simulink library "Microgrid_Library"  (R2020a)
settings: Solver=VariableStepAuto  StartTime=0.0  StopTime=10.0  RelTol=1e-3
libraries: simulink x6944, powerlib x1090, simulink_extras x40, powerlib_meascontrol x20
(part 1 of 77)
settings Model: Creator=burmesdani  LastModifiedBy=Daniel
settings DataIOCC: ReturnWorkspaceOutputs=off
settings DebuggingCC: ArithmeticOperatorsInVariantConditions=warning
settings SFSimCC: SimUseLocalCustomCode=off  CustomCodeUndefinedFunction=UseInterfaceOnly
settings RTWCC: RTWUseLocalCustomCode=off

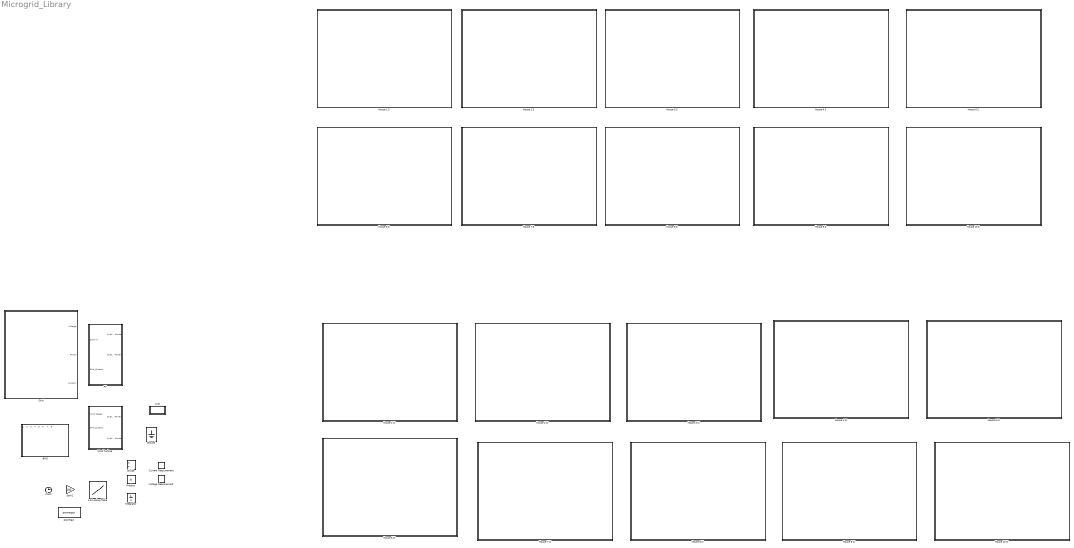
== system Microgrid_Library ==
  1-D Lookup Table : Lookup_n-D  NumberOfTableDimensions=1  BreakpointsForDimension1=[0 1 2 3 4 5 6 7 8 9 10 11 12 13 14 15 16 17 18 19 20 21 22 23]  Table=|  InternalRulePriority=Speed  RndMeth=Simplest  InputSameDT=on
      | [0
      | 0
      | 0
      | 0
      | 0
      | 0
      | 0
      | 0
      | 11
      | 36
      | 116
      | 198
      | 386
      | 372
      | 294
      | 178
      | 31
      | 0
      | 0
      | 0
      | 0
      | 0
      | 0
      | 0]
  BMS : Subsystem  mask{v=240, c=25}
      mask prompts: v "Voltage (V)"; c "Capacity (Ah)"
  Clock : Clock
  Current Measurement : powerlib/Measurements/Current Measurement  PhasorSimulation=off  OutputType=Complex  PSBequivalent=0
  Divide : Product  Inputs=*/
  Gain1 : Gain  Gain=100
  Grid : Subsystem  mask{v=240}
      mask prompts: v "Voltage"
      mask Display: image('power_pole_2.jpg');
  Ground : powerlib/Elements/Ground  LConnTagsString=a
  House 1 S : Subsystem  mask{V=240, t=60, K_L1_W=18, K_L1_On_1=0, K_L1_Off_1=27000, K_L1_On_2=73800, K_L1_Off_2=86400, K_L2_W=100, K_L2_On_1=61200, K_L2_Off_1=63000, Fridge_W=150, T_Initial=1, T_Low=1, T_High=2, Jug_W=2000, Jug_On_1=23400, Jug_On_2=61200, Jug_On_3=72000, Toaster_W=1100, Toaster_On_1=27000, Toaster_On_2=0, N_Elements=3, Oven_On_1=61200, Oven_Off_1=64800, Shower_On_a=69000, Shower_L_a=300, Shower_On_b=70200, Shower_L_b=300, Shower_On_c=76200, Shower_L_c=180, K_L3_W=40, K_L3_On_1=66600, K_L3_Off_1=69300, K_L3_On_2=79200, K_L3_Off_2=82800, HW_W=2000, M=135, D=0.488, L=1.335, Ti=7, Ta=20, T_Low_WH=57, T_High_WH=62.55, Clock_1_W=5, Lamp_1_W=25, L1_On_1=72600, L1_Off_1=82800, L1_On_2=0, L1_Off_2=0, K_L4_W=18, K_L4_On_1=72000, K_L4_Off_1=72600, Clock_2_W=3, Lamp_2_W=40, L2_On_1=25200, L2_Off_1=27000, L2_On_2=75600, L2_Off_2=82800, K_L5_W=40, K_L5_On_1=25200, K_L5_Off_1=27000, K_L5_On_2=75600, K_L5_Off_2=82800, Phone_C_1_W=5, Phone_C_1_On_a=0, Phone_C_1_Off_a=29700, Phone_C_1_On_b=79200, Phone_C_1_Off_b=86400, Phone_C_2_W=5, Phone_C_2_On_a=0, Phone_C_2_Off_a=29700, Phone_C_2_On_b=82800, Phone_C_2_Off_b=86400, Phone_C_3_W=5, Phone_C_3_On_a=0, Phone_C_3_Off_a=29700, Phone_C_3_On_b=82800, Phone_C_3_Off_b=86400, Laptop_C_1_W=65, Laptop_C_1_On_a=64800, Laptop_C_1_Off_a=72900, Laptop_C_2_W=60, Laptop_C_2_On_a=0, Laptop_C_2_Off_a=29700, Laptop_C_2_On_b=82800, Laptop_C_2_Off_b=86400, Lamp_3_W=40, L3_On_1=21600, L3_Off_1=27000, L3_On_2=72000, L3_Off_2=82800, K_L6_W=120, K_L6_On_1=68400, K_L6_Off_1=70200, Heater_W=2100, Heater_On_1=63660, Heater_Off_1=69240, Stereo_W=50, Stereo_On_1=57600, Stereo_Off_1=61500, TV_W=400, TV_On_1=21600, TV_Off_1=26400, TV_On_2=59400, TV_Off_2=79200}
      mask prompts: V "Voltage"; t "Time"; K_L1_W "Light_1_W"; K_L1_On_1 "Light_1_On_a"; K_L1_Off_1 "Light_1_Off_a"; K_L1_On_2 "Light_1_On_b"; K_L1_Off_2 "Light_1_Off_b"; K_L2_W "Light_2_W"; K_L2_On_1 "Light_2_On_a"; K_L2_Off_1 "Light_2_Off_a"; T_Initial "Fridge_Initial_Temp"; T_Low "Fridge_T_Low"; T_High "Fridge_T_High"; N_Elements "Oven_#_Elements"; Shower_L_a "Shower_Length_a"; Shower_L_b "Shower_Length_b"; Shower_L_c "Shower_Length_c"; K_L3_W "Light_3_W"; K_L3_On_1 "Light_3_On_a"; K_L3_Off_1 "Light_3_Off_a"; K_L3_On_2 "Light_3_On_b"; K_L3_Off_2 "Light_3_Off_b"; M "Mass of water"; D "HW_Diameter"; L "HW_Length"; Ti "Inlet Temp"; Ta "Ambient Temp"; L1_On_1 "Lamp_1_On_1"; L1_Off_1 "Lamp_1_Off_1"; L1_On_2 "Lamp_1_On_2"; L1_Off_2 "Lamp_1_Off_2"; K_L4_W "Light_4_W"; K_L4_On_1 "Light_4_On_a"; K_L4_Off_1 "Light_4_Off_a"; L2_On_1 "Lamp_2_On_1"; L2_Off_1 "Lamp_2_Off_1"; L2_On_2 "Lamp_2_On_2"; L2_Off_2 "Lamp_2_Off_2"; K_L5_W "Light_5_W"; K_L5_On_1 "Light_5_On_a"; K_L5_Off_1 "Light_5_Off_a"; K_L5_On_2 "Light_5_On_b"; K_L5_Off_2 "Light_5_Off_b"; L3_On_1 "Lamp_3_On_1"; L3_Off_1 "Lamp_3_Off_1"; L3_On_2 "Lamp_3_On_2"; L3_Off_2 "Lamp_3_Off_2"; K_L6_W "Light_6_W"; K_L6_On_1 "Light_6_On_a"; K_L6_Off_1 "Light_6_Off_a"
      mask DialogControl Container4 Prompt: House 1
      mask DialogControl Container10 Prompt: Kitchen
      mask DialogControl Container22 Prompt: Bathroom
      mask DialogControl Container20 Prompt: Bedroom 1
      mask DialogControl Container16 Prompt: Bedroom 2
      mask DialogControl Container11 Prompt: Lounge
      mask Display: image('house.jpg');
  House 1 W : Subsystem  mask{V=240, t=60, K_L1_W=18, K_L1_On_1=0, K_L1_Off_1=27000, K_L1_On_2=64800, K_L1_Off_2=86400, K_L2_W=100, K_L2_On_1=61200, K_L2_Off_1=63000, Fridge_W=150, T_Initial=1, T_Low=1, T_High=2, Jug_W=2000, Jug_On_1=23400, Jug_On_2=61200, Jug_On_3=72000, Toaster_W=1100, Toaster_On_1=27000, Toaster_On_2=0, N_Elements=3, Oven_On_1=61200, Oven_Off_1=64800, Shower_On_a=69000, Shower_L_a=300, Shower_On_b=70200, Shower_L_b=300, Shower_On_c=76200, Shower_L_c=180, K_L3_W=40, K_L3_On_1=66600, K_L3_Off_1=69300, K_L3_On_2=79200, K_L3_Off_2=82800, HW_W=2000, M=135, D=0.488, L=1.335, Ti=7, Ta=20, T_Low_WH=57, T_High_WH=62.55, Clock_1_W=5, Lamp_1_W=25, L1_On_1=70200, L1_Off_1=82800, L1_On_2=0, L1_Off_2=0, K_L4_W=18, K_L4_On_1=64800, K_L4_Off_1=70200, Clock_2_W=3, Lamp_2_W=40, L2_On_1=25200, L2_Off_1=27000, L2_On_2=75600, L2_Off_2=82800, K_L5_W=40, K_L5_On_1=25200, K_L5_Off_1=27000, K_L5_On_2=75600, K_L5_Off_2=82800, Phone_C_1_W=5, Phone_C_1_On_a=0, Phone_C_1_Off_a=29700, Phone_C_1_On_b=79200, Phone_C_1_Off_b=86400, Phone_C_2_W=5, Phone_C_2_On_a=0, Phone_C_2_Off_a=29700, Phone_C_2_On_b=82800, Phone_C_2_Off_b=86400, Phone_C_3_W=5, Phone_C_3_On_a=0, Phone_C_3_Off_a=29700, Phone_C_3_On_b=82800, Phone_C_3_Off_b=86400, Laptop_C_1_W=65, Laptop_C_1_On_a=64800, Laptop_C_1_Off_a=72900, Laptop_C_2_W=60, Laptop_C_2_On_a=0, Laptop_C_2_Off_a=29700, Laptop_C_2_On_b=82800, Laptop_C_2_Off_b=86400, Lamp_3_W=40, L3_On_1=21600, L3_Off_1=27000, L3_On_2=64800, L3_Off_2=68400, K_L6_W=120, K_L6_On_1=68400, K_L6_Off_1=70200, Heater_W=2100, Heater_On_1=63660, Heater_Off_1=69240, Stereo_W=50, Stereo_On_1=57600, Stereo_Off_1=61500, TV_W=400, TV_On_1=21600, TV_Off_1=26400, TV_On_2=59400, TV_Off_2=79200}
      mask prompts: V "Voltage"; t "Time"; K_L1_W "Light_1_W"; K_L1_On_1 "Light_1_On_a"; K_L1_Off_1 "Light_1_Off_a"; K_L1_On_2 "Light_1_On_b"; K_L1_Off_2 "Light_1_Off_b"; K_L2_W "Light_2_W"; K_L2_On_1 "Light_2_On_a"; K_L2_Off_1 "Light_2_Off_a"; T_Initial "Fridge_Initial_Temp"; T_Low "Fridge_T_Low"; T_High "Fridge_T_High"; N_Elements "Oven_#_Elements"; Shower_L_a "Shower_Length_a"; Shower_L_b "Shower_Length_b"; Shower_L_c "Shower_Length_c"; K_L3_W "Light_3_W"; K_L3_On_1 "Light_3_On_a"; K_L3_Off_1 "Light_3_Off_a"; K_L3_On_2 "Light_3_On_b"; K_L3_Off_2 "Light_3_Off_b"; M "Mass of water"; D "HW_Diameter"; L "HW_Length"; Ti "Inlet Temp"; Ta "Ambient Temp"; L1_On_1 "Lamp_1_On_1"; L1_Off_1 "Lamp_1_Off_1"; L1_On_2 "Lamp_1_On_2"; L1_Off_2 "Lamp_1_Off_2"; K_L4_W "Light_4_W"; K_L4_On_1 "Light_4_On_a"; K_L4_Off_1 "Light_4_Off_a"; L2_On_1 "Lamp_2_On_1"; L2_Off_1 "Lamp_2_Off_1"; L2_On_2 "Lamp_2_On_2"; L2_Off_2 "Lamp_2_Off_2"; K_L5_W "Light_5_W"; K_L5_On_1 "Light_5_On_a"; K_L5_Off_1 "Light_5_Off_a"; K_L5_On_2 "Light_5_On_b"; K_L5_Off_2 "Light_5_Off_b"; L3_On_1 "Lamp_3_On_1"; L3_Off_1 "Lamp_3_Off_1"; L3_On_2 "Lamp_3_On_2"; L3_Off_2 "Lamp_3_Off_2"; K_L6_W "Light_6_W"; K_L6_On_1 "Light_6_On_a"; K_L6_Off_1 "Light_6_Off_a"
      mask DialogControl Container4 Prompt: House 1
      mask DialogControl Container10 Prompt: Kitchen
      mask DialogControl Container22 Prompt: Bathroom
      mask DialogControl Container20 Prompt: Bedroom 1
      mask DialogControl Container16 Prompt: Bedroom 2
      mask DialogControl Container11 Prompt: Lounge
      mask Display: image('house.jpg');
  House 10 S : Subsystem  mask{V=240, t=60, K_L1_W=100, K_L1_On_1=25920, K_L1_Off_1=26940, K_L1_On_2=72120, K_L1_Off_2=75900, K_L2_W=40, K_L2_On_1=71820, K_L2_Off_1=73440, Fridge_W=150, T_Initial=1.2, T_Low=1, T_High=2.8, Jug_W=2000, Jug_On_1=26940, Jug_On_2=70980, Jug_On_3=0, Toaster_W=450, Toaster_On_1=25500, Toaster_On_2=0, N_Elements=2, Oven_On_1=66180, Oven_Off_1=67560, Shower_On_a=79680, Shower_L_a=420, Shower_On_b=80400, Shower_L_b=240, Shower_On_c=0, Shower_L_c=0, K_L3_W=24, K_L3_On_1=79200, K_L3_Off_1=80700, K_L3_On_2=0, K_L3_Off_2=0, HW_W=2000, M=135, D=0.488, L=1.335, Ti=7, Ta=20, T_Low_WH=58, T_High_WH=63.8, Clock_1_W=0, Lamp_1_W=1, L1_On_1=0, L1_Off_1=0, L1_On_2=0, L1_Off_2=0, K_L4_W=1, K_L4_On_1=0, K_L4_Off_1=0, Clock_2_W=5, Lamp_2_W=18, L2_On_1=25680, L2_Off_1=27600, L2_On_2=83940, L2_Off_2=85020, K_L5_W=40, K_L5_On_1=26400, K_L5_Off_1=27780, K_L5_On_2=81000, K_L5_Off_2=83940, Phone_C_1_W=7, Phone_C_1_On_a=0, Phone_C_1_Off_a=27300, Phone_C_1_On_b=79200, Phone_C_1_Off_b=86400, Phone_C_2_W=0, Phone_C_2_On_a=0, Phone_C_2_Off_a=0, Phone_C_2_On_b=0, Phone_C_2_Off_b=0, Phone_C_3_W=5, Phone_C_3_On_a=0, Phone_C_3_Off_a=27300, Phone_C_3_On_b=83940, Phone_C_3_Off_b=86400, Laptop_C_1_W=35, Laptop_C_1_On_a=63900, Laptop_C_1_Off_a=75600, Laptop_C_2_W=65, Laptop_C_2_On_a=0, Laptop_C_2_Off_a=21600, Laptop_C_2_On_b=66600, Laptop_C_2_Off_b=86400, Lamp_3_W=36, L3_On_1=27000, L3_Off_1=27600, L3_On_2=72600, L3_Off_2=81000, K_L6_W=24, K_L6_On_1=72000, K_L6_Off_1=75000, Heater_W=2000, Heater_On_1=65100, Heater_Off_1=69000, Stereo_W=1, Stereo_On_1=0, Stereo_Off_1=0, TV_W=200, TV_On_1=66300, TV_Off_1=83940, TV_On_2=0, TV_Off_2=0}
      mask prompts: V "Voltage"; t "Time"; K_L1_W "Light_1_W"; K_L1_On_1 "Light_1_On_a"; K_L1_Off_1 "Light_1_Off_a"; K_L1_On_2 "Light_1_On_b"; K_L1_Off_2 "Light_1_Off_b"; K_L2_W "Light_2_W"; K_L2_On_1 "Light_2_On_a"; K_L2_Off_1 "Light_2_Off_a"; T_Initial "Fridge_Initial_Temp"; T_Low "Fridge_T_Low"; T_High "Fridge_T_High"; N_Elements "Oven_#_Elements"; Shower_L_a "Shower_Length_a"; Shower_L_b "Shower_Length_b"; Shower_L_c "Shower_Length_c"; K_L3_W "Light_3_W"; K_L3_On_1 "Light_3_On_a"; K_L3_Off_1 "Light_3_Off_a"; K_L3_On_2 "Light_3_On_b"; K_L3_Off_2 "Light_3_Off_b"; M "Mass of water"; D "HW_Diameter"; L "HW_Length"; Ti "Inlet Temp"; Ta "Ambient Temp"; L1_On_1 "Lamp_1_On_1"; L1_Off_1 "Lamp_1_Off_1"; L1_On_2 "Lamp_1_On_2"; L1_Off_2 "Lamp_1_Off_2"; K_L4_W "Light_4_W"; K_L4_On_1 "Light_4_On_a"; K_L4_Off_1 "Light_4_Off_a"; L2_On_1 "Lamp_2_On_1"; L2_Off_1 "Lamp_2_Off_1"; L2_On_2 "Lamp_2_On_2"; L2_Off_2 "Lamp_2_Off_2"; K_L5_W "Light_5_W"; K_L5_On_1 "Light_5_On_a"; K_L5_Off_1 "Light_5_Off_a"; K_L5_On_2 "Light_5_On_b"; K_L5_Off_2 "Light_5_Off_b"; L3_On_1 "Lamp_3_On_1"; L3_Off_1 "Lamp_3_Off_1"; L3_On_2 "Lamp_3_On_2"; L3_Off_2 "Lamp_3_Off_2"; K_L6_W "Light_6_W"; K_L6_On_1 "Light_6_On_a"; K_L6_Off_1 "Light_6_Off_a"
      mask DialogControl Container4 Prompt: House 1
      mask DialogControl Container10 Prompt: Kitchen
      mask DialogControl Container22 Prompt: Bathroom
      mask DialogControl Container20 Prompt: Bedroom 1
      mask DialogControl Container16 Prompt: Bedroom 2
      mask DialogControl Container11 Prompt: Lounge
      mask Display: image('house.jpg');
  House 10 W : Subsystem  mask{V=240, t=60, K_L1_W=100, K_L1_On_1=25920, K_L1_Off_1=26940, K_L1_On_2=66120, K_L1_Off_2=69900, K_L2_W=40, K_L2_On_1=65820, K_L2_Off_1=67440, Fridge_W=150, T_Initial=1.2, T_Low=1, T_High=2.8, Jug_W=2000, Jug_On_1=26940, Jug_On_2=70980, Jug_On_3=0, Toaster_W=450, Toaster_On_1=25500, Toaster_On_2=0, N_Elements=2, Oven_On_1=66180, Oven_Off_1=67560, Shower_On_a=79680, Shower_L_a=420, Shower_On_b=80400, Shower_L_b=240, Shower_On_c=0, Shower_L_c=0, K_L3_W=24, K_L3_On_1=79200, K_L3_Off_1=80700, K_L3_On_2=0, K_L3_Off_2=0, HW_W=2000, M=135, D=0.488, L=1.335, Ti=7, Ta=20, T_Low_WH=58, T_High_WH=63.8, Clock_1_W=0, Lamp_1_W=1, L1_On_1=0, L1_Off_1=0, L1_On_2=0, L1_Off_2=0, K_L4_W=1, K_L4_On_1=0, K_L4_Off_1=0, Clock_2_W=5, Lamp_2_W=18, L2_On_1=25680, L2_Off_1=27600, L2_On_2=83940, L2_Off_2=85020, K_L5_W=40, K_L5_On_1=26400, K_L5_Off_1=27780, K_L5_On_2=81000, K_L5_Off_2=83940, Phone_C_1_W=7, Phone_C_1_On_a=0, Phone_C_1_Off_a=27300, Phone_C_1_On_b=79200, Phone_C_1_Off_b=86400, Phone_C_2_W=0, Phone_C_2_On_a=0, Phone_C_2_Off_a=0, Phone_C_2_On_b=0, Phone_C_2_Off_b=0, Phone_C_3_W=5, Phone_C_3_On_a=0, Phone_C_3_Off_a=27300, Phone_C_3_On_b=83940, Phone_C_3_Off_b=86400, Laptop_C_1_W=35, Laptop_C_1_On_a=63900, Laptop_C_1_Off_a=75600, Laptop_C_2_W=65, Laptop_C_2_On_a=0, Laptop_C_2_Off_a=21600, Laptop_C_2_On_b=66600, Laptop_C_2_Off_b=86400, Lamp_3_W=36, L3_On_1=27000, L3_Off_1=27600, L3_On_2=66600, L3_Off_2=81000, K_L6_W=24, K_L6_On_1=63000, K_L6_Off_1=66000, Heater_W=2000, Heater_On_1=65100, Heater_Off_1=69000, Stereo_W=1, Stereo_On_1=0, Stereo_Off_1=0, TV_W=200, TV_On_1=66300, TV_Off_1=83940, TV_On_2=0, TV_Off_2=0}
      mask prompts: V "Voltage"; t "Time"; K_L1_W "Light_1_W"; K_L1_On_1 "Light_1_On_a"; K_L1_Off_1 "Light_1_Off_a"; K_L1_On_2 "Light_1_On_b"; K_L1_Off_2 "Light_1_Off_b"; K_L2_W "Light_2_W"; K_L2_On_1 "Light_2_On_a"; K_L2_Off_1 "Light_2_Off_a"; T_Initial "Fridge_Initial_Temp"; T_Low "Fridge_T_Low"; T_High "Fridge_T_High"; N_Elements "Oven_#_Elements"; Shower_L_a "Shower_Length_a"; Shower_L_b "Shower_Length_b"; Shower_L_c "Shower_Length_c"; K_L3_W "Light_3_W"; K_L3_On_1 "Light_3_On_a"; K_L3_Off_1 "Light_3_Off_a"; K_L3_On_2 "Light_3_On_b"; K_L3_Off_2 "Light_3_Off_b"; M "Mass of water"; D "HW_Diameter"; L "HW_Length"; Ti "Inlet Temp"; Ta "Ambient Temp"; L1_On_1 "Lamp_1_On_1"; L1_Off_1 "Lamp_1_Off_1"; L1_On_2 "Lamp_1_On_2"; L1_Off_2 "Lamp_1_Off_2"; K_L4_W "Light_4_W"; K_L4_On_1 "Light_4_On_a"; K_L4_Off_1 "Light_4_Off_a"; L2_On_1 "Lamp_2_On_1"; L2_Off_1 "Lamp_2_Off_1"; L2_On_2 "Lamp_2_On_2"; L2_Off_2 "Lamp_2_Off_2"; K_L5_W "Light_5_W"; K_L5_On_1 "Light_5_On_a"; K_L5_Off_1 "Light_5_Off_a"; K_L5_On_2 "Light_5_On_b"; K_L5_Off_2 "Light_5_Off_b"; L3_On_1 "Lamp_3_On_1"; L3_Off_1 "Lamp_3_Off_1"; L3_On_2 "Lamp_3_On_2"; L3_Off_2 "Lamp_3_Off_2"; K_L6_W "Light_6_W"; K_L6_On_1 "Light_6_On_a"; K_L6_Off_1 "Light_6_Off_a"
      mask DialogControl Container4 Prompt: House 1
      mask DialogControl Container10 Prompt: Kitchen
      mask DialogControl Container22 Prompt: Bathroom
      mask DialogControl Container20 Prompt: Bedroom 1
      mask DialogControl Container16 Prompt: Bedroom 2
      mask DialogControl Container11 Prompt: Lounge
      mask Display: image('house.jpg');
  House 2 S : Subsystem  mask{V=240, t=60, K_L1_W=40, K_L1_On_1=75000, K_L1_Off_1=84600, K_L1_On_2=0, K_L1_Off_2=0, Dishwasher_W=1200, Dishwasher_On=72000, Fridge_W=150, T_Initial=1.9, T_Low=1, T_High=2.4, Jug_W=1500, Jug_On_1=25200, Jug_On_2=64200, Jug_On_3=0, Toaster_W=900, Toaster_On_1=23400, Toaster_On_2=27120, N_Elements=4, Oven_On_1=63600, Oven_Off_1=67200, Shower_On_a=21720, Shower_L_a=240, Shower_On_b=23640, Shower_L_b=300, Shower_On_c=0, Shower_L_c=0, K_L3_W=60, K_L3_On_1=21400, K_L3_Off_1=22140, K_L3_On_2=23580, K_L3_Off_2=24780, HW_W=2000, M=177, D=0.488, L=1.72, Ti=7, Ta=20, T_Low_WH=58, T_High_WH=61, Clock_1_W=5, Lamp_1_W=18, L1_On_1=79740, L1_Off_1=83040, L1_On_2=0, L1_Off_2=0, K_L4_W=24, K_L4_On_1=21000, K_L4_Off_1=27360, Clock_2_W=6, Lamp_2_W=24, L2_On_1=26820, L2_Off_1=29160, L2_On_2=73800, L2_Off_2=76800, K_L5_W=40, K_L5_On_1=28800, K_L5_Off_1=30240, K_L5_On_2=72300, K_L5_Off_2=83880, Phone_C_1_W=3, Phone_C_1_On_a=0, Phone_C_1_Off_a=35700, Phone_C_1_On_b=76800, Phone_C_1_Off_b=86400, Phone_C_2_W=7, Phone_C_2_On_a=0, Phone_C_2_Off_a=35760, Phone_C_2_On_b=76800, Phone_C_2_Off_b=86400, Phone_C_3_W=5, Phone_C_3_On_a=0, Phone_C_3_Off_a=35700, Phone_C_3_On_b=77040, Phone_C_3_Off_b=86400, Laptop_C_1_W=55, Laptop_C_1_On_a=76800, Laptop_C_1_Off_a=86400, Laptop_C_2_W=65, Laptop_C_2_On_a=0, Laptop_C_2_Off_a=28500, Laptop_C_2_On_b=77160, Laptop_C_2_Off_b=86400, Lamp_3_W=60, L3_On_1=21600, L3_Off_1=27000, L3_On_2=74760, L3_Off_2=83220, K_L6_W=60, K_L6_On_1=74400, K_L6_Off_1=76200, Heater_W=1800, Heater_On_1=62040, Heater_Off_1=68640, Stereo_W=100, Stereo_On_1=42480, Stereo_Off_1=58200, TV_W=500, TV_On_1=21900, TV_Off_1=26580, TV_On_2=63000, TV_Off_2=73200}
      mask prompts: V "Voltage"; t "Time"; K_L1_W "Light_1_W"; K_L1_On_1 "Light_1_On_a"; K_L1_Off_1 "Light_1_Off_a"; K_L1_On_2 "Light_1_On_b"; K_L1_Off_2 "Light_1_Off_b"; T_Initial "Fridge_Initial_Temp"; T_Low "Fridge_T_Low"; T_High "Fridge_T_High"; N_Elements "Oven_#_Elements"; Shower_L_a "Shower_Length_a"; Shower_L_b "Shower_Length_b"; Shower_L_c "Shower_Length_c"; K_L3_W "Light_3_W"; K_L3_On_1 "Light_3_On_a"; K_L3_Off_1 "Light_3_Off_a"; K_L3_On_2 "Light_3_On_b"; K_L3_Off_2 "Light_3_Off_b"; M "Mass of water"; D "HW_Diameter"; L "HW_Length"; Ti "Inlet Temp"; Ta "Ambient Temp"; L1_On_1 "Lamp_1_On_1"; L1_Off_1 "Lamp_1_Off_1"; L1_On_2 "Lamp_1_On_2"; L1_Off_2 "Lamp_1_Off_2"; K_L4_W "Light_4_W"; K_L4_On_1 "Light_4_On_a"; K_L4_Off_1 "Light_4_Off_a"; L2_On_1 "Lamp_2_On_1"; L2_Off_1 "Lamp_2_Off_1"; L2_On_2 "Lamp_2_On_2"; L2_Off_2 "Lamp_2_Off_2"; K_L5_W "Light_5_W"; K_L5_On_1 "Light_5_On_a"; K_L5_Off_1 "Light_5_Off_a"; K_L5_On_2 "Light_5_On_b"; K_L5_Off_2 "Light_5_Off_b"; L3_On_1 "Lamp_3_On_1"; L3_Off_1 "Lamp_3_Off_1"; L3_On_2 "Lamp_3_On_2"; L3_Off_2 "Lamp_3_Off_2"; K_L6_W "Light_6_W"; K_L6_On_1 "Light_6_On_a"; K_L6_Off_1 "Light_6_Off_a"
      mask DialogControl Container4 Prompt: House 1
      mask DialogControl Container10 Prompt: Kitchen
      mask DialogControl Container22 Prompt: Bathroom
      mask DialogControl Container20 Prompt: Bedroom 1
      mask DialogControl Container16 Prompt: Bedroom 2
      mask DialogControl Container11 Prompt: Lounge
      mask Display: image('house.jpg');
  House 2 W : Subsystem  mask{V=240, t=60, K_L1_W=40, K_L1_On_1=63000, K_L1_Off_1=72600, K_L1_On_2=0, K_L1_Off_2=0, Dishwasher_W=1200, Dishwasher_On=72000, Fridge_W=150, T_Initial=1.9, T_Low=1, T_High=2.4, Jug_W=1500, Jug_On_1=25200, Jug_On_2=64200, Jug_On_3=0, Toaster_W=900, Toaster_On_1=23400, Toaster_On_2=27120, N_Elements=4, Oven_On_1=63600, Oven_Off_1=67200, Shower_On_a=21720, Shower_L_a=240, Shower_On_b=23640, Shower_L_b=300, Shower_On_c=0, Shower_L_c=0, K_L3_W=60, K_L3_On_1=21480, K_L3_Off_1=22140, K_L3_On_2=23580, K_L3_Off_2=24780, HW_W=2000, M=177, D=0.488, L=1.72, Ti=7, Ta=20, T_Low_WH=58, T_High_WH=61, Clock_1_W=5, Lamp_1_W=18, L1_On_1=79740, L1_Off_1=83040, L1_On_2=0, L1_Off_2=0, K_L4_W=24, K_L4_On_1=21000, K_L4_Off_1=27360, Clock_2_W=6, Lamp_2_W=24, L2_On_1=26820, L2_Off_1=29160, L2_On_2=73800, L2_Off_2=76800, K_L5_W=40, K_L5_On_1=28800, K_L5_Off_1=30240, K_L5_On_2=72300, K_L5_Off_2=83880, Phone_C_1_W=3, Phone_C_1_On_a=0, Phone_C_1_Off_a=35700, Phone_C_1_On_b=76800, Phone_C_1_Off_b=86400, Phone_C_2_W=7, Phone_C_2_On_a=0, Phone_C_2_Off_a=35760, Phone_C_2_On_b=76800, Phone_C_2_Off_b=86400, Phone_C_3_W=5, Phone_C_3_On_a=0, Phone_C_3_Off_a=35700, Phone_C_3_On_b=77040, Phone_C_3_Off_b=86400, Laptop_C_1_W=55, Laptop_C_1_On_a=76800, Laptop_C_1_Off_a=86400, Laptop_C_2_W=65, Laptop_C_2_On_a=0, Laptop_C_2_Off_a=28500, Laptop_C_2_On_b=77160, Laptop_C_2_Off_b=86400, Lamp_3_W=60, L3_On_1=21600, L3_Off_1=27000, L3_On_2=62760, L3_Off_2=83220, K_L6_W=60, K_L6_On_1=68400, K_L6_Off_1=70200, Heater_W=1800, Heater_On_1=62040, Heater_Off_1=68640, Stereo_W=100, Stereo_On_1=42480, Stereo_Off_1=58200, TV_W=500, TV_On_1=21900, TV_Off_1=26580, TV_On_2=63000, TV_Off_2=73200}
      mask prompts: V "Voltage"; t "Time"; K_L1_W "Light_1_W"; K_L1_On_1 "Light_1_On_a"; K_L1_Off_1 "Light_1_Off_a"; K_L1_On_2 "Light_1_On_b"; K_L1_Off_2 "Light_1_Off_b"; T_Initial "Fridge_Initial_Temp"; T_Low "Fridge_T_Low"; T_High "Fridge_T_High"; N_Elements "Oven_#_Elements"; Shower_L_a "Shower_Length_a"; Shower_L_b "Shower_Length_b"; Shower_L_c "Shower_Length_c"; K_L3_W "Light_3_W"; K_L3_On_1 "Light_3_On_a"; K_L3_Off_1 "Light_3_Off_a"; K_L3_On_2 "Light_3_On_b"; K_L3_Off_2 "Light_3_Off_b"; M "Mass of water"; D "HW_Diameter"; L "HW_Length"; Ti "Inlet Temp"; Ta "Ambient Temp"; L1_On_1 "Lamp_1_On_1"; L1_Off_1 "Lamp_1_Off_1"; L1_On_2 "Lamp_1_On_2"; L1_Off_2 "Lamp_1_Off_2"; K_L4_W "Light_4_W"; K_L4_On_1 "Light_4_On_a"; K_L4_Off_1 "Light_4_Off_a"; L2_On_1 "Lamp_2_On_1"; L2_Off_1 "Lamp_2_Off_1"; L2_On_2 "Lamp_2_On_2"; L2_Off_2 "Lamp_2_Off_2"; K_L5_W "Light_5_W"; K_L5_On_1 "Light_5_On_a"; K_L5_Off_1 "Light_5_Off_a"; K_L5_On_2 "Light_5_On_b"; K_L5_Off_2 "Light_5_Off_b"; L3_On_1 "Lamp_3_On_1"; L3_Off_1 "Lamp_3_Off_1"; L3_On_2 "Lamp_3_On_2"; L3_Off_2 "Lamp_3_Off_2"; K_L6_W "Light_6_W"; K_L6_On_1 "Light_6_On_a"; K_L6_Off_1 "Light_6_Off_a"
      mask DialogControl Container4 Prompt: House 1
      mask DialogControl Container10 Prompt: Kitchen
      mask DialogControl Container22 Prompt: Bathroom
      mask DialogControl Container20 Prompt: Bedroom 1
      mask DialogControl Container16 Prompt: Bedroom 2
      mask DialogControl Container11 Prompt: Lounge
      mask Display: image('house.jpg');
  House 3 S : Subsystem  mask{V=240, t=60, K_L1_W=24, K_L1_On_1=21600, K_L1_Off_1=26580, K_L1_On_2=75000, K_L1_Off_2=79020, K_L2_W=60, K_L2_On_1=75000, K_L2_Off_1=80400, Fridge_W=200, T_Initial=1.5, T_Low=1.5, T_High=2.65, Jug_W=2000, Jug_On_1=21720, Jug_On_2=43560, Jug_On_3=73800, Toaster_W=1200, Toaster_On_1=25260, Toaster_On_2=28920, N_Elements=1, Oven_On_1=58680, Oven_Off_1=68760, Shower_On_a=70440, Shower_L_a=360, Shower_On_b=76740, Shower_L_b=180, Shower_On_c=81000, Shower_L_c=180, K_L3_W=80, K_L3_On_1=70200, K_L3_Off_1=70800, K_L3_On_2=76320, K_L3_Off_2=81300, HW_W=3000, M=250, D=0.58, L=1.56, Ti=7, Ta=20, T_Low_WH=59, T_High_WH=61, Clock_1_W=6, Lamp_1_W=18, L1_On_1=77400, L1_Off_1=81120, L1_On_2=0, L1_Off_2=0, K_L4_W=60, K_L4_On_1=75600, K_L4_Off_1=77400, Clock_2_W=4, Lamp_2_W=24, L2_On_1=84120, L2_Off_1=86880, L2_On_2=0, L2_Off_2=0, K_L5_W=40, K_L5_On_1=25320, K_L5_Off_1=28980, K_L5_On_2=81000, K_L5_Off_2=84180, Phone_C_1_W=7, Phone_C_1_On_a=0, Phone_C_1_Off_a=27300, Phone_C_1_On_b=79200, Phone_C_1_Off_b=86400, Phone_C_2_W=6, Phone_C_2_On_a=0, Phone_C_2_Off_a=27300, Phone_C_2_On_b=79200, Phone_C_2_Off_b=86400, Phone_C_3_W=5, Phone_C_3_On_a=0, Phone_C_3_Off_a=0, Phone_C_3_On_b=0, Phone_C_3_Off_b=0, Laptop_C_1_W=1, Laptop_C_1_On_a=0, Laptop_C_1_Off_a=0, Laptop_C_2_W=45, Laptop_C_2_On_a=0, Laptop_C_2_Off_a=6000, Laptop_C_2_On_b=66600, Laptop_C_2_Off_b=86400, Lamp_3_W=40, L3_On_1=24900, L3_Off_1=30600, L3_On_2=72000, L3_Off_2=81000, K_L6_W=60, K_L6_On_1=74760, K_L6_Off_1=77520, Heater_W=1500, Heater_On_1=64800, Heater_Off_1=78000, Stereo_W=1, Stereo_On_1=0, Stereo_Off_1=0, TV_W=150, TV_On_1=25200, TV_Off_1=28800, TV_On_2=72240, TV_Off_2=81000}
      mask prompts: V "Voltage"; t "Time"; K_L1_W "Light_1_W"; K_L1_On_1 "Light_1_On_a"; K_L1_Off_1 "Light_1_Off_a"; K_L1_On_2 "Light_1_On_b"; K_L1_Off_2 "Light_1_Off_b"; K_L2_W "Light_2_W"; K_L2_On_1 "Light_2_On_a"; K_L2_Off_1 "Light_2_Off_a"; T_Initial "Fridge_Initial_Temp"; T_Low "Fridge_T_Low"; T_High "Fridge_T_High"; N_Elements "Oven_#_Elements"; Shower_L_a "Shower_Length_a"; Shower_L_b "Shower_Length_b"; Shower_L_c "Shower_Length_c"; K_L3_W "Light_3_W"; K_L3_On_1 "Light_3_On_a"; K_L3_Off_1 "Light_3_Off_a"; K_L3_On_2 "Light_3_On_b"; K_L3_Off_2 "Light_3_Off_b"; M "Mass of water"; D "HW_Diameter"; L "HW_Length"; Ti "Inlet Temp"; Ta "Ambient Temp"; L1_On_1 "Lamp_1_On_1"; L1_Off_1 "Lamp_1_Off_1"; L1_On_2 "Lamp_1_On_2"; L1_Off_2 "Lamp_1_Off_2"; K_L4_W "Light_4_W"; K_L4_On_1 "Light_4_On_a"; K_L4_Off_1 "Light_4_Off_a"; L2_On_1 "Lamp_2_On_1"; L2_Off_1 "Lamp_2_Off_1"; L2_On_2 "Lamp_2_On_2"; L2_Off_2 "Lamp_2_Off_2"; K_L5_W "Light_5_W"; K_L5_On_1 "Light_5_On_a"; K_L5_Off_1 "Light_5_Off_a"; K_L5_On_2 "Light_5_On_b"; K_L5_Off_2 "Light_5_Off_b"; L3_On_1 "Lamp_3_On_1"; L3_Off_1 "Lamp_3_Off_1"; L3_On_2 "Lamp_3_On_2"; L3_Off_2 "Lamp_3_Off_2"; K_L6_W "Light_6_W"; K_L6_On_1 "Light_6_On_a"; K_L6_Off_1 "Light_6_Off_a"
      mask DialogControl Container4 Prompt: House 1
      mask DialogControl Container10 Prompt: Kitchen
      mask DialogControl Container22 Prompt: Bathroom
      mask DialogControl Container20 Prompt: Bedroom 1
      mask DialogControl Container16 Prompt: Bedroom 2
      mask DialogControl Container11 Prompt: Lounge
      mask Display: image('house.jpg');
  House 3 W : Subsystem  mask{V=240, t=60, K_L1_W=24, K_L1_On_1=21600, K_L1_Off_1=26580, K_L1_On_2=63000, K_L1_Off_2=68400, K_L2_W=60, K_L2_On_1=63000, K_L2_Off_1=68400, Fridge_W=200, T_Initial=1.5, T_Low=1.5, T_High=2.65, Jug_W=2000, Jug_On_1=21720, Jug_On_2=43560, Jug_On_3=73800, Toaster_W=1200, Toaster_On_1=25260, Toaster_On_2=28920, N_Elements=1, Oven_On_1=58680, Oven_Off_1=68760, Shower_On_a=70440, Shower_L_a=360, Shower_On_b=76740, Shower_L_b=180, Shower_On_c=81000, Shower_L_c=180, K_L3_W=80, K_L3_On_1=70200, K_L3_Off_1=70800, K_L3_On_2=76320, K_L3_Off_2=81300, HW_W=3000, M=250, D=0.58, L=1.56, Ti=7, Ta=20, T_Low_WH=59, T_High_WH=61, Clock_1_W=6, Lamp_1_W=18, L1_On_1=71400, L1_Off_1=81120, L1_On_2=0, L1_Off_2=0, K_L4_W=60, K_L4_On_1=69600, K_L4_Off_1=71400, Clock_2_W=4, Lamp_2_W=24, L2_On_1=84120, L2_Off_1=86880, L2_On_2=0, L2_Off_2=0, K_L5_W=40, K_L5_On_1=25320, K_L5_Off_1=28980, K_L5_On_2=81000, K_L5_Off_2=84180, Phone_C_1_W=7, Phone_C_1_On_a=0, Phone_C_1_Off_a=27300, Phone_C_1_On_b=79200, Phone_C_1_Off_b=86400, Phone_C_2_W=6, Phone_C_2_On_a=0, Phone_C_2_Off_a=27300, Phone_C_2_On_b=79200, Phone_C_2_Off_b=86400, Phone_C_3_W=5, Phone_C_3_On_a=0, Phone_C_3_Off_a=0, Phone_C_3_On_b=0, Phone_C_3_Off_b=0, Laptop_C_1_W=1, Laptop_C_1_On_a=0, Laptop_C_1_Off_a=0, Laptop_C_2_W=45, Laptop_C_2_On_a=0, Laptop_C_2_Off_a=6000, Laptop_C_2_On_b=66600, Laptop_C_2_Off_b=86400, Lamp_3_W=40, L3_On_1=24900, L3_Off_1=30600, L3_On_2=72000, L3_Off_2=81000, K_L6_W=60, K_L6_On_1=68760, K_L6_Off_1=71520, Heater_W=1500, Heater_On_1=64800, Heater_Off_1=78000, Stereo_W=1, Stereo_On_1=0, Stereo_Off_1=0, TV_W=150, TV_On_1=25200, TV_Off_1=28800, TV_On_2=72240, TV_Off_2=81000}
      mask prompts: V "Voltage"; t "Time"; K_L1_W "Light_1_W"; K_L1_On_1 "Light_1_On_a"; K_L1_Off_1 "Light_1_Off_a"; K_L1_On_2 "Light_1_On_b"; K_L1_Off_2 "Light_1_Off_b"; K_L2_W "Light_2_W"; K_L2_On_1 "Light_2_On_a"; K_L2_Off_1 "Light_2_Off_a"; T_Initial "Fridge_Initial_Temp"; T_Low "Fridge_T_Low"; T_High "Fridge_T_High"; N_Elements "Oven_#_Elements"; Shower_L_a "Shower_Length_a"; Shower_L_b "Shower_Length_b"; Shower_L_c "Shower_Length_c"; K_L3_W "Light_3_W"; K_L3_On_1 "Light_3_On_a"; K_L3_Off_1 "Light_3_Off_a"; K_L3_On_2 "Light_3_On_b"; K_L3_Off_2 "Light_3_Off_b"; M "Mass of water"; D "HW_Diameter"; L "HW_Length"; Ti "Inlet Temp"; Ta "Ambient Temp"; L1_On_1 "Lamp_1_On_1"; L1_Off_1 "Lamp_1_Off_1"; L1_On_2 "Lamp_1_On_2"; L1_Off_2 "Lamp_1_Off_2"; K_L4_W "Light_4_W"; K_L4_On_1 "Light_4_On_a"; K_L4_Off_1 "Light_4_Off_a"; L2_On_1 "Lamp_2_On_1"; L2_Off_1 "Lamp_2_Off_1"; L2_On_2 "Lamp_2_On_2"; L2_Off_2 "Lamp_2_Off_2"; K_L5_W "Light_5_W"; K_L5_On_1 "Light_5_On_a"; K_L5_Off_1 "Light_5_Off_a"; K_L5_On_2 "Light_5_On_b"; K_L5_Off_2 "Light_5_Off_b"; L3_On_1 "Lamp_3_On_1"; L3_Off_1 "Lamp_3_Off_1"; L3_On_2 "Lamp_3_On_2"; L3_Off_2 "Lamp_3_Off_2"; K_L6_W "Light_6_W"; K_L6_On_1 "Light_6_On_a"; K_L6_Off_1 "Light_6_Off_a"
      mask DialogControl Container4 Prompt: House 1
      mask DialogControl Container10 Prompt: Kitchen
      mask DialogControl Container22 Prompt: Bathroom
      mask DialogControl Container20 Prompt: Bedroom 1
      mask DialogControl Container16 Prompt: Bedroom 2
      mask DialogControl Container11 Prompt: Lounge
      mask Display: image('house.jpg');
  House 4 S : Subsystem  mask{V=240, t=60, K_L1_W=60, K_L1_On_1=75180, K_L1_Off_1=76200, K_L1_On_2=0, K_L1_Off_2=0, K_L2_W=1, K_L2_On_1=0, K_L2_Off_1=0, Fridge_W=150, T_Initial=1, T_Low=1, T_High=3.6, Jug_W=1800, Jug_On_1=23760, Jug_On_2=79800, Jug_On_3=0, Toaster_W=1000, Toaster_On_1=21600, Toaster_On_2=0, N_Elements=2, Oven_On_1=75240, Oven_Off_1=76140, Shower_On_a=20040, Shower_L_a=180, Shower_On_b=0, Shower_L_b=0, Shower_On_c=0, Shower_L_c=0, K_L3_W=40, K_L3_On_1=19800, K_L3_Off_1=20400, K_L3_On_2=0, K_L3_Off_2=0, HW_W=2000, M=88, D=0.488, L=0.955, Ti=7, Ta=20, T_Low_WH=55, T_High_WH=59.5, Clock_1_W=0, Lamp_1_W=1, L1_On_1=0, L1_Off_1=0, L1_On_2=0, L1_Off_2=0, K_L4_W=1, K_L4_On_1=0, K_L4_Off_1=0, Clock_2_W=4, Lamp_2_W=40, L2_On_1=19680, L2_Off_1=21600, L2_On_2=82800, L2_Off_2=84600, K_L5_W=1, K_L5_On_1=0, K_L5_Off_1=0, K_L5_On_2=0, K_L5_Off_2=0, Phone_C_1_W=5, Phone_C_1_On_a=0, Phone_C_1_Off_a=27300, Phone_C_1_On_b=79200, Phone_C_1_Off_b=86400, Phone_C_2_W=0, Phone_C_2_On_a=0, Phone_C_2_Off_a=0, Phone_C_2_On_b=0, Phone_C_2_Off_b=0, Phone_C_3_W=0, Phone_C_3_On_a=0, Phone_C_3_Off_a=0, Phone_C_3_On_b=0, Phone_C_3_Off_b=0, Laptop_C_1_W=35, Laptop_C_1_On_a=63900, Laptop_C_1_Off_a=75600, Laptop_C_2_W=65, Laptop_C_2_On_a=0, Laptop_C_2_Off_a=21600, Laptop_C_2_On_b=66600, Laptop_C_2_Off_b=86400, Lamp_3_W=18, L3_On_1=72000, L3_Off_1=82800, L3_On_2=0, L3_Off_2=0, K_L6_W=40, K_L6_On_1=75000, K_L6_Off_1=78000, Heater_W=500, Heater_On_1=65100, Heater_Off_1=82200, Stereo_W=100, Stereo_On_1=68640, Stereo_Off_1=70740, TV_W=300, TV_On_1=70800, TV_Off_1=82800, TV_On_2=0, TV_Off_2=0}
      mask prompts: V "Voltage"; t "Time"; K_L1_W "Light_1_W"; K_L1_On_1 "Light_1_On_a"; K_L1_Off_1 "Light_1_Off_a"; K_L1_On_2 "Light_1_On_b"; K_L1_Off_2 "Light_1_Off_b"; K_L2_W "Light_2_W"; K_L2_On_1 "Light_2_On_a"; K_L2_Off_1 "Light_2_Off_a"; T_Initial "Fridge_Initial_Temp"; T_Low "Fridge_T_Low"; T_High "Fridge_T_High"; N_Elements "Oven_#_Elements"; Shower_L_a "Shower_Length_a"; Shower_L_b "Shower_Length_b"; Shower_L_c "Shower_Length_c"; K_L3_W "Light_3_W"; K_L3_On_1 "Light_3_On_a"; K_L3_Off_1 "Light_3_Off_a"; K_L3_On_2 "Light_3_On_b"; K_L3_Off_2 "Light_3_Off_b"; M "Mass of water"; D "HW_Diameter"; L "HW_Length"; Ti "Inlet Temp"; Ta "Ambient Temp"; L1_On_1 "Lamp_1_On_1"; L1_Off_1 "Lamp_1_Off_1"; L1_On_2 "Lamp_1_On_2"; L1_Off_2 "Lamp_1_Off_2"; K_L4_W "Light_4_W"; K_L4_On_1 "Light_4_On_a"; K_L4_Off_1 "Light_4_Off_a"; L2_On_1 "Lamp_2_On_1"; L2_Off_1 "Lamp_2_Off_1"; L2_On_2 "Lamp_2_On_2"; L2_Off_2 "Lamp_2_Off_2"; K_L5_W "Light_5_W"; K_L5_On_1 "Light_5_On_a"; K_L5_Off_1 "Light_5_Off_a"; K_L5_On_2 "Light_5_On_b"; K_L5_Off_2 "Light_5_Off_b"; L3_On_1 "Lamp_3_On_1"; L3_Off_1 "Lamp_3_Off_1"; L3_On_2 "Lamp_3_On_2"; L3_Off_2 "Lamp_3_Off_2"; K_L6_W "Light_6_W"; K_L6_On_1 "Light_6_On_a"; K_L6_Off_1 "Light_6_Off_a"
      mask DialogControl Container4 Prompt: House 1
      mask DialogControl Container10 Prompt: Kitchen
      mask DialogControl Container22 Prompt: Bathroom
      mask DialogControl Container20 Prompt: Bedroom 1
      mask DialogControl Container16 Prompt: Bedroom 2
      mask DialogControl Container11 Prompt: Lounge
      mask Display: image('house.jpg');
  House 4 W : Subsystem  mask{V=240, t=60, K_L1_W=60, K_L1_On_1=69180, K_L1_Off_1=70200, K_L1_On_2=0, K_L1_Off_2=0, K_L2_W=1, K_L2_On_1=0, K_L2_Off_1=0, Fridge_W=150, T_Initial=1, T_Low=1, T_High=3.6, Jug_W=1800, Jug_On_1=23760, Jug_On_2=79800, Jug_On_3=0, Toaster_W=1000, Toaster_On_1=21600, Toaster_On_2=0, N_Elements=2, Oven_On_1=69240, Oven_Off_1=70140, Shower_On_a=20040, Shower_L_a=180, Shower_On_b=0, Shower_L_b=0, Shower_On_c=0, Shower_L_c=0, K_L3_W=40, K_L3_On_1=19800, K_L3_Off_1=20400, K_L3_On_2=0, K_L3_Off_2=0, HW_W=2000, M=88, D=0.488, L=0.955, Ti=7, Ta=20, T_Low_WH=55, T_High_WH=59.5, Clock_1_W=0, Lamp_1_W=1, L1_On_1=0, L1_Off_1=0, L1_On_2=0, L1_Off_2=0, K_L4_W=1, K_L4_On_1=0, K_L4_Off_1=0, Clock_2_W=4, Lamp_2_W=40, L2_On_1=19680, L2_Off_1=21600, L2_On_2=82800, L2_Off_2=84600, K_L5_W=1, K_L5_On_1=0, K_L5_Off_1=0, K_L5_On_2=0, K_L5_Off_2=0, Phone_C_1_W=5, Phone_C_1_On_a=0, Phone_C_1_Off_a=27300, Phone_C_1_On_b=79200, Phone_C_1_Off_b=86400, Phone_C_2_W=0, Phone_C_2_On_a=0, Phone_C_2_Off_a=0, Phone_C_2_On_b=0, Phone_C_2_Off_b=0, Phone_C_3_W=0, Phone_C_3_On_a=0, Phone_C_3_Off_a=0, Phone_C_3_On_b=0, Phone_C_3_Off_b=0, Laptop_C_1_W=35, Laptop_C_1_On_a=63900, Laptop_C_1_Off_a=75600, Laptop_C_2_W=65, Laptop_C_2_On_a=0, Laptop_C_2_Off_a=21600, Laptop_C_2_On_b=66600, Laptop_C_2_Off_b=86400, Lamp_3_W=18, L3_On_1=66000, L3_Off_1=82800, L3_On_2=0, L3_Off_2=0, K_L6_W=40, K_L6_On_1=63000, K_L6_Off_1=66000, Heater_W=2000, Heater_On_1=65100, Heater_Off_1=82200, Stereo_W=100, Stereo_On_1=68640, Stereo_Off_1=70740, TV_W=300, TV_On_1=70800, TV_Off_1=82800, TV_On_2=0, TV_Off_2=0}
      mask prompts: V "Voltage"; t "Time"; K_L1_W "Light_1_W"; K_L1_On_1 "Light_1_On_a"; K_L1_Off_1 "Light_1_Off_a"; K_L1_On_2 "Light_1_On_b"; K_L1_Off_2 "Light_1_Off_b"; K_L2_W "Light_2_W"; K_L2_On_1 "Light_2_On_a"; K_L2_Off_1 "Light_2_Off_a"; T_Initial "Fridge_Initial_Temp"; T_Low "Fridge_T_Low"; T_High "Fridge_T_High"; N_Elements "Oven_#_Elements"; Shower_L_a "Shower_Length_a"; Shower_L_b "Shower_Length_b"; Shower_L_c "Shower_Length_c"; K_L3_W "Light_3_W"; K_L3_On_1 "Light_3_On_a"; K_L3_Off_1 "Light_3_Off_a"; K_L3_On_2 "Light_3_On_b"; K_L3_Off_2 "Light_3_Off_b"; M "Mass of water"; D "HW_Diameter"; L "HW_Length"; Ti "Inlet Temp"; Ta "Ambient Temp"; L1_On_1 "Lamp_1_On_1"; L1_Off_1 "Lamp_1_Off_1"; L1_On_2 "Lamp_1_On_2"; L1_Off_2 "Lamp_1_Off_2"; K_L4_W "Light_4_W"; K_L4_On_1 "Light_4_On_a"; K_L4_Off_1 "Light_4_Off_a"; L2_On_1 "Lamp_2_On_1"; L2_Off_1 "Lamp_2_Off_1"; L2_On_2 "Lamp_2_On_2"; L2_Off_2 "Lamp_2_Off_2"; K_L5_W "Light_5_W"; K_L5_On_1 "Light_5_On_a"; K_L5_Off_1 "Light_5_Off_a"; K_L5_On_2 "Light_5_On_b"; K_L5_Off_2 "Light_5_Off_b"; L3_On_1 "Lamp_3_On_1"; L3_Off_1 "Lamp_3_Off_1"; L3_On_2 "Lamp_3_On_2"; L3_Off_2 "Lamp_3_Off_2"; K_L6_W "Light_6_W"; K_L6_On_1 "Light_6_On_a"; K_L6_Off_1 "Light_6_Off_a"